simulink model "ROMmodel_C1"  (R2023a)
settings: Solver=ode15s  StartTime=0.0  StopTime=1  RelTol=1e-3
model workspace (MAT data in the model file):
  AbdFlex.InterfaceFrameOrigins = [9.55660193925e-12  0.0959385649697  0.873753152305; 0  0.141459732056  1.16; 0  0.0965046510696  0.917910743713; 0  0.107959739685  0.951083679199; 0  0.115579937935  0.987707740784; 0  0.115174944878  1.02503444672; 0  0.108694094658  1.05941337585]
  AbdFlex.InterfaceFrameOrientations = [0.845190937114  -0.534464479475  0  0; 1  0  0  0; 0.984100197668  -0.177614191295  0  0; 0.981622160978  -0.190834831927  0  0; 0.996532605642  -0.0832031603563  0  0; 0.99971936632  0.0236894196747  0  0; 0.994308117365  0.106542797703  0  0]
  AbdFlex.StiffnessMatrix = [43361.7570519 -71.0199455086 224.354982647 0.636554401137 1595.02399491 -409.551381431 1442.61743363 0.240122053479 21.2711338415 -1.01415324364 563.926777958 237.112118311 ... (1936 elements, 44x44)]
  AbdFlex.MassMatrix = [0.803313954226 0.00157932528245 -0.000141409930888 -6.06093264666e-05 -0.0137464920365 -0.0376787188144 0.0264567110796 0.000154097209329 -0.000731720801434 4.72355458836e-05 -0.0189804601472 -0.0220920974904 ... (1936 elements, 44x44)]
  AbdFlex.GraphicVertexCoordinates = [-0.0249230308533 -0.0239328804016 -0.0255591678619 -0.0472221679687 -0.0482721481323 -0.0496672782898 0.0537743339539 0.053651309967 0.0579661331177 0.00969921779633 0.00303560614586 0.0045106883049 ... (24849 elements, 8283x3)]
  AbdFlex.GraphicFacetVertexIndices = [1 4 7 10 13 16 19 22 25 28 31 34 ... (49686 elements, 16562x3)]
  AbdFlex.GraphicReconstructionMatrix = [-0.0338257388504 0.0155859996042 -0.0281553023712 -0.0314821376202 0.0122493157715 -0.022038070746 -0.0338754415912 0.0120802860785 -0.0268680028964 0.000241192212069 0.0929827996464 -0.0670573257083 ... (1093356 elements, 24849x44)]
  C_IVD = 0.05
  E_AbdFlex = 0.126
  E_IVD1 = 2.1
  E_IVD2 = 2.2
  E_IVD3 = 1.1
  E_IVD4 = 1.7
  E_IVD5 = 3.2
  E_IVD_Optimization : sldodialogs.data.SessionData (value not decoded)
  E_TLFflex = 18.8
  FollowerLoad = 0
  IAP_Pa = 0
  IAP_activation_AbdFlex = 1
  ISL_color = [1 1 1]
  ITL_color = [1 1 1]
  IVD1.InterfaceFrameOrigins = [0.000134062032297  0.0934932940185  1.0361888027; 0.000751265379852  0.0916872213141  1.04242465264]
  IVD1.InterfaceFrameOrientations = [0.998042258458  0.062435296582  -0.00366490237461  -0.000229268114415; 0.994308029006  0.106542788235  0.000419180698234  4.49163428851e-05]
  IVD1.StiffnessMatrix = [58604.0590509 224.692445169 -1325.81371063 11.5597748027 178.44666362 120.502622484 -58604.0590509 -224.69244516 1325.81371063 -10.5664072195 187.81775116 -14.520750133 ... (484 elements, 22x22)]
  IVD1.MassMatrix = [0.00273222555649 2.66777679307e-05 -0.000140988898492 -4.00936120478e-08 -1.20933230549e-06 -3.51698017382e-06 0.00135518459087 -2.57456518557e-05 0.000137551041775 6.02428528449e-08 5.38698497169e-07 -2.32643510543e-06 ... (484 elements, 22x22)]
  IVD1.GraphicVertexCoordinates = [0.0211187839508 0.0199944629669 0.0202089264725 0.0199764213562 0.0218328495026 0.0198232516565 0.019585439682 0.0207308521271 0.0184082126617 0.0186618622796 0.0138851182479 0.018299514437 ... (3174 elements, 1058x3)]
  IVD1.GraphicFacetVertexIndices = [1 4 5 1 8 11 14 17 20 23 26 29 ... (6336 elements, 2112x3)]
  IVD1.GraphicReconstructionMatrix = [0.256410242145 -0.0564519072051 0.218397018955 0.0410678719594 -0.0182233415057 -0.0032254600483 0.0910430192482 -0.0254706457501 0.0263929427812 0.0147481346277 0.0132702076923 0.00432342515969 ... (69828 elements, 3174x22)]
  IVD2.InterfaceFrameOrigins = [0.000686098346355  0.0963570089985  1.0036271702; 0.000140868100677  0.0958234446692  1.01049438711]
  IVD2.InterfaceFrameOrientations = [0.999634432353  -0.0270344006557  -0.000377796940173  1.02172489428e-05; 0.999691730803  0.0236887648214  0.00743335453971  0.000176141286458]
  IVD2.StiffnessMatrix = [56957.407386 -78.2947242504 2352.16731735 -17.870981357 205.105453917 67.0078916822 -56957.407386 78.2947242326 -2352.16731734 17.1536156345 187.3158902 -36.5747621608 ... (484 elements, 22x22)]
  IVD2.MassMatrix = [0.00289222462833 -1.63017581735e-06 -0.000121359011889 5.79569398759e-07 -1.89296391846e-06 -4.55924182037e-06 0.00149269437853 1.27567995597e-06 0.00011497170797 -3.47796966863e-07 -3.11083563762e-07 -3.41019744486e-06 ... (484 elements, 22x22)]
  IVD2.GraphicVertexCoordinates = [0.00590759760452 0.00585390377045 0.00765654303278 0.00585360622406 0.00761780643463 0.00650919151306 -0.0178935775757 -0.0169768047333 -0.0178932800293 -0.0166804516015 0.0200935579478 0.0162637276088 ... (3231 elements, 1077x3)]
  IVD2.GraphicFacetVertexIndices = [1 4 6 7 10 11 14 17 15 22 25 28 ... (6450 elements, 2150x3)]
  IVD2.GraphicReconstructionMatrix = [0 0 0 0 0 0 0 0 0 0.00543110901344 0.00220321782751 -0.000251171527064 ... (71082 elements, 3231x22)]
  IVD3.InterfaceFrameOrigins = [0.000574607735293  0.0917086135207  0.970572111233; 0.000722363834978  0.0932279951662  0.976368556268]
  IVD3.InterfaceFrameOrientations = [0.988186593281  -0.153247753692  -0.00152541347062  0.000236560776491; 0.996532076153  -0.0832031161482  0.00102728042444  -8.57703776093e-05]
  IVD3.StiffnessMatrix = [71304.4780645 62.9837567154 443.050268141 -9.93169999493 256.42256406 64.3509875002 -71304.4780645 -62.9837567061 -443.050268129 10.2397805565 156.824460393 -172.680396477 ... (484 elements, 22x22)]
  IVD3.MassMatrix = [0.00260198817992 -2.70630834152e-05 -0.000116699850829 4.26142700457e-07 -2.89748765883e-06 -5.58917800559e-06 0.00134564423394 2.80094300316e-05 0.000115788329975 -4.23251433064e-07 -1.01057781469e-06 -3.70278721028e-06 ... (484 elements, 22x22)]
  IVD3.GraphicVertexCoordinates = [0.0185354701694 0.0186535515176 0.0152037481739 0.0123575800793 0.0160170742583 -0.0196599748606 -0.0204075508118 -0.0210658721924 -0.00348978233337 -0.00541844558716 -0.00541840410233 0.0207612419128 ... (3219 elements, 1073x3)]
  IVD3.GraphicFacetVertexIndices = [1 3 6 9 12 15 15 19 22 19 26 29 ... (6426 elements, 2142x3)]
  IVD3.GraphicReconstructionMatrix = [0 0 0 0 0 0 0 0 0 0 0 0 ... (70818 elements, 3219x22)]
  IVD4.InterfaceFrameOrigins = [0.000825538133572  0.0809240019453  0.938796986262; 0.000702816801697  0.0842740141936  0.946273348843]
  IVD4.InterfaceFrameOrientations = [0.982784432836  -0.18463310027  0.0066204169361  -0.00124376014022; 0.98161854002  -0.190834127986  -0.00266623568967  0.000518336545308]
  IVD4.StiffnessMatrix = [51887.5634084 863.844390995 1885.99958486 3.3949859294 210.302792214 -101.202544563 -51887.5634085 -863.844390999 -1885.99958487 -3.53527809988 177.858897611 -72.7274405247 ... (484 elements, 22x22)]
  IVD4.MassMatrix = [0.00383476851166 0.000110815534324 0.000274865642717 -1.01453683106e-07 -1.40266595323e-06 1.05697122783e-06 0.00193817912343 -0.00010255217908 -0.000262363391912 1.30237786765e-07 -7.38115151254e-07 4.90959424197e-07 ... (484 elements, 22x22)]
  IVD4.GraphicVertexCoordinates = [-0.00692420768738 -0.00905326843262 -0.00795169639587 0.00511226844788 0.00382958173752 0.00571388053894 0.00635967063904 0.00634820652008 0.00634847593307 0.0207787151337 0.021058713913 0.0196662540436 ... (3462 elements, 1154x3)]
  IVD4.GraphicFacetVertexIndices = [1 4 7 10 13 16 19 22 25 28 31 33 ... (6912 elements, 2304x3)]
  IVD4.GraphicReconstructionMatrix = [0.770337775779 -0.00512670557356 -0.011312131418 0.762650254966 -0.0169645937534 -0.0232414027029 0.996499969395 6.68963973463e-05 -0.000139362803193 0.268412218038 0.0114672418521 0.0636586351525 ... (76164 elements, 3462x22)]
  IVD5.InterfaceFrameOrigins = [0.000376663432785  0.0678773637683  0.904322521845; 0.000371714158635  0.0728931824713  0.914631727172]
  IVD5.InterfaceFrameOrientations = [0.963982062514  -0.265850048581  -0.0076111073418  0.00209901546435; 0.970389572461  -0.241521069818  0.00331223233616  -0.000824384267944]
  IVD5.StiffnessMatrix = [35645.3690223 -248.67083816 -709.947740413 9.43031031091 176.709163243 -84.3584941894 -35645.3690223 248.670838177 709.947740436 -10.4276807361 190.762751227 -94.4309836875 ... (484 elements, 22x22)]
  IVD5.MassMatrix = [0.00473206856693 -8.93206818325e-05 -0.000159656867099 -3.06552159693e-07 -3.25796315086e-07 -2.69868887073e-06 0.00266836567966 8.98622650209e-05 0.000153831007551 3.02118872769e-07 -6.97732994802e-06 7.09837910569e-08 ... (484 elements, 22x22)]
  IVD5.GraphicVertexCoordinates = [0.00965603343984 0.00946946966218 0.00773647180015 -0.000354907704017 0.000199558018267 0.00273271407381 -0.0111632396442 -0.00971436501195 -0.0129904044408 -0.00470198234377 -0.00657061876603 -0.0080616476784 ... (3822 elements, 1274x3)]
  IVD5.GraphicFacetVertexIndices = [1 4 7 10 13 16 19 21 24 26 29 32 ... (7632 elements, 2544x3)]
  IVD5.GraphicReconstructionMatrix = [0 0 0 0 0 0 0 0 0 0 0 0 ... (84084 elements, 3822x22)]
  Left_EO = 0
  Left_IO = 0
  Left_Lat = 18
  Left_LongThor = 0
  Left_MF = 0
  Left_Psoas = 0
  Left_RA = 0
  Moment_AR = 0
  Moment_FE = 7.5
  Moment_LB = 0
  ROM_draft1_sdosession : sldodialogs.data.SessionData (value not decoded)
  ROM_draft3_sdosession : sldodialogs.data.SessionData (value not decoded)
  Right_EO = 0
  Right_IO = 0
  Right_Lat = 18
  Right_LongThor = 0
  Right_MF = 0
  Right_Psoas = 0
  Right_RA = 0
  SSL_color = [1 1 1]
  TLFflex.InterfaceFrameOrigins = [0  0.0286700446606  1.0249522934; 0  0.0299802474976  1.00330503082; 0  0.0254783229828  0.97747177887; 0  0.0207425162792  0.956563842773; 0  0.0144365491867  0.93638368988; 0.049806123159  0.0172518861278  0.922800188881; -0.049806123159  0.0172518861278  0.922800188881; 0.0340383493  -0.010672627765  1.12996748354; 0.0388434691641  -0.00188389440244  1.08212073323; 0.0590475906704  0.0162601057615  1.03264080317; -0.0340383493  -0.010672627765  1.12996748354; -0.0388434691641  -0.00188389440244  1.08212073323; -0.0590475906704  0.0162601057615  1.03264080317]
  TLFflex.InterfaceFrameOrientations = [1  0  0  0; 1  0  0  0; 1  0  0  0; 1  0  0  0; 1  0  0  0; 1  0  0  0; 1  0  0  0; 1  0  0  0; 1  0  0  0; 1  0  0  0; 1  0  0  0; 1  0  0  0; 1  0  0  0]
  TLFflex.StiffnessMatrix = [3066.73590221 18.8871843042 -24.6815054809 0.185355906935 -27.1562999474 -5.00119068721 -2890.55682783 -19.4003823931 24.0128755145 0.225494141951 -25.823043414 2.53663392749 ... (7744 elements, 88x88)]
  TLFflex.MassMatrix = [0.00625522807647 9.49097881264e-06 4.16499662158e-06 -9.78079002209e-08 5.18483232966e-05 -9.11375657364e-07 0.000159214873984 -2.78264603728e-06 -8.02480617259e-06 4.32112453313e-09 2.74154275053e-06 6.17256747742e-06 ... (7744 elements, 88x88)]
  TLFflex.GraphicVertexCoordinates = [-0.0377008171739 -0.0393963165839 -0.0371501493438 0.0614751544791 0.062221073601 0.064095957414 -0.0512719215728 -0.0484790364525 -0.0501779383507 0.0308447883186 0.0321367582765 0.034096923506 ... (26841 elements, 8947x3)]
  TLFflex.GraphicFacetVertexIndices = [1 4 7 10 13 16 19 22 25 28 28 22 ... (53670 elements, 17890x3)]
  TLFflex.GraphicReconstructionMatrix = [0.000464639411671 -0.0178024512743 0.0479271668365 -0.0147231027484 -0.0347333700407 0.045198070726 -0.011349330195 -0.0316574127367 0.0470820475173 -0.00429611905822 0.00365635322465 -0.00297745351186 ... (2362008 elements, 26841x88)]
  TLFflex2.InterfaceFrameOrigins = [0  0.0286700446606  1.0249522934; 0  0.0299802474976  1.00330503082; 0  0.0254783229828  0.97747177887; 0  0.0207425162792  0.956563842773; 0  0.0144365491867  0.93638368988; 0.049806123159  0.0172518861278  0.922800188881; -0.049806123159  0.0172518861278  0.922800188881; 0.0340383493  -0.010672627765  1.12996748354; 0.0388434691641  -0.00188389440244  1.08212073323; 0.0590475906704  0.0162601057615  1.03264080317; -0.0340383493  -0.010672627765  1.12996748354; -0.0388434691641  -0.00188389440244  1.08212073323; -0.0590475906704  0.0162601057615  1.03264080317]
  TLFflex2.InterfaceFrameOrientations = [1  0  0  0; 1  0  0  0; 1  0  0  0; 1  0  0  0; 1  0  0  0; 1  0  0  0; 1  0  0  0; 1  0  0  0; 1  0  0  0; 1  0  0  0; 1  0  0  0; 1  0  0  0; 1  0  0  0]
  TLFflex2.StiffnessMatrix = [3098.16338957 1.85271730265 -2.49344260049 0.0117348050607 -27.4580802755 -4.86875385453 -2927.62857118 -1.89895408806 1.79381618556 0.0403210517395 -26.3931492349 2.34539218598 ... (7744 elements, 88x88)]
  TLFflex2.MassMatrix = [0.00647785700791 -7.69135453041e-06 1.52474405977e-05 -1.66463703749e-08 5.42797116484e-05 -4.88742850574e-07 0.000137311871526 -3.29576441791e-06 3.78371240816e-06 4.33544974799e-09 2.96751088594e-06 6.68238191038e-06 ... (7744 elements, 88x88)]
  TLFflex2.GraphicVertexCoordinates = [0.0187781253155 0.0208486402039 0.0186395397985 0.00960678133698 0.00986427632128 0.0093318262088 0.0122389386043 0.0589722700755 0.0600399895232 0.0577259274432 0.059047073965 0.0568156199971 ... (22956 elements, 7652x3)]
  TLFflex2.GraphicFacetVertexIndices = [1 4 7 8 11 14 17 20 23 26 29 32 ... (45900 elements, 15300x3)]
  TLFflex2.GraphicReconstructionMatrix = [-0.0992460193127 -0.842553190279 -0.169575794245 -0.10048467577 -0.840523805717 -0.167598295688 -0.0880669070773 -0.863197887424 -0.169707154947 -0.0115968837044 0.017512943826 -0.0284024768683 ... (2020128 elements, 22956x88)]
  eo_color = [1 0 0]
  io_color = [0 1 0]
  latissimus_color = [0 0.5 1]
  ligament_radius = 0.5
  longissimus_color = [1 0 1]
  multifidus_color = [0 1 1]
  muscle_radius = 1
  psoas_color = [0 0 1]
  ra_color = [1 1 0.5]
libraries: checkpacklib x11, simulink_extras x9, simulink x2
settings Model: Creator=siril  LastModifiedBy=siril
settings DataIOCC: SaveFormat=StructureWithTime  ReturnWorkspaceOutputsName=SDOSimTest_Log
settings DebuggingCC: UniqueDataStoreMsg=error
settings SimscapeCC: SimscapeLogType=all

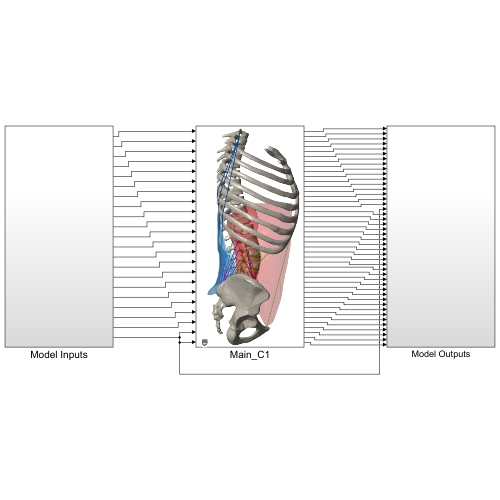
[thumbnail: Simulink's stored preview of the top-level diagram]
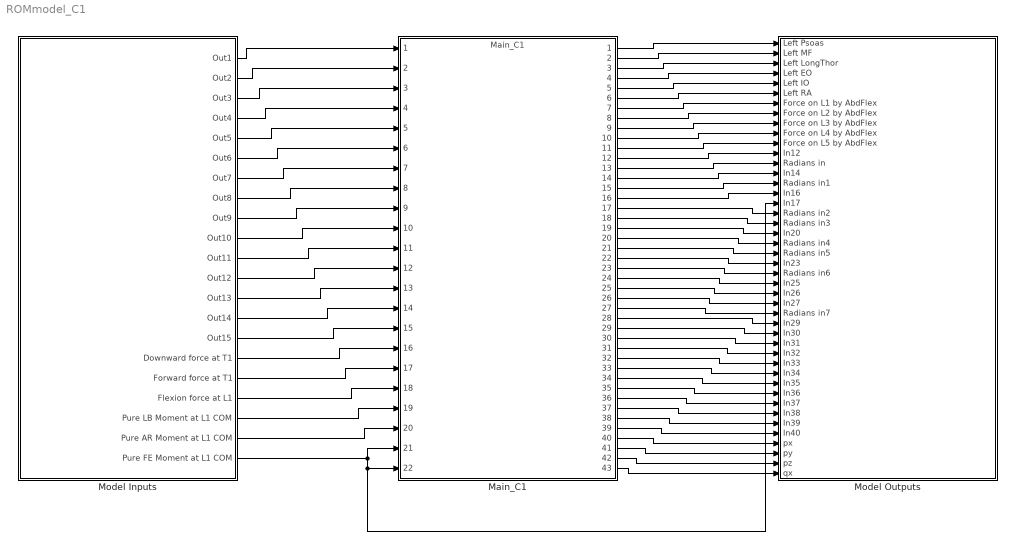
== system ROMmodel_C1 ==
  Main_C1 : ModelReference  ModelNameDialog=Main_C1.slxp  ModelReferenceVersion=1.132  CopyOfModelProtected=on
      mask Display: image('$imagefile'); % Use "Edit Image" on toolstrip to change.
  Model Inputs : Subsystem
  Model Outputs : Subsystem
  wires:
  Main_C1/32 -> Model Outputs/33(In33)
  Main_C1/31 -> Model Outputs/32(In32)
  Model Inputs/20(Pure AR Moment at L1 COM) -> Main_C1/20
  Model Inputs/1(Out1) -> Main_C1/1
  Model Inputs/7(Out7) -> Main_C1/7
  Model Inputs/8(Out8) -> Main_C1/8
  Model Inputs/3(Out3) -> Main_C1/3
  Main_C1/20 -> Model Outputs/21(Radians in4)
  Model Inputs/2(Out2) -> Main_C1/2
  Main_C1/24 -> Model Outputs/25(In25)
  Main_C1/23 -> Model Outputs/24(Radians in6)
  Model Inputs/12(Out12) -> Main_C1/12
  Model Inputs/4(Out4) -> Main_C1/4
  Main_C1/13 -> Model Outputs/13(Radians in)
  Model Inputs/5(Out5) -> Main_C1/5
  Model Inputs/6(Out6) -> Main_C1/6
  Model Inputs/9(Out9) -> Main_C1/9
  Main_C1/19 -> Model Outputs/20(In20)
  Model Inputs/10(Out10) -> Main_C1/10
  Main_C1/22 -> Model Outputs/23(In23)
  Model Inputs/11(Out11) -> Main_C1/11
  Main_C1/25 -> Model Outputs/26(In26)
  Model Inputs/14(Out14) -> Main_C1/14
  Model Inputs/13(Out13) -> Main_C1/13
  Main_C1/26 -> Model Outputs/27(In27)
  Model Inputs/15(Out15) -> Main_C1/15
  Main_C1/28 -> Model Outputs/29(In29)
  Main_C1/27 -> Model Outputs/28(Radians in7)
  Model Inputs/16(Downward force at T1) -> Main_C1/16
  Main_C1/29 -> Model Outputs/30(In30)
  Model Inputs/18(Flexion force at L1) -> Main_C1/18
  Model Inputs/17(Forward force at T1) -> Main_C1/17
  Main_C1/30 -> Model Outputs/31(In31)
  Model Inputs/19(Pure LB Moment at L1 COM) -> Main_C1/19
  Main_C1/33 -> Model Outputs/34(In34)
  Model Inputs/21(Pure FE Moment at L1 COM) -> Model Outputs/17(In17), Main_C1/22, Main_C1/21
  Main_C1/1 -> Model Outputs/1(Left Psoas)
  Main_C1/2 -> Model Outputs/2(Left MF)
  Main_C1/3 -> Model Outputs/3(Left LongThor)
  Main_C1/4 -> Model Outputs/4(Left EO)
  Main_C1/5 -> Model Outputs/5(Left IO)
  Main_C1/6 -> Model Outputs/6(Left RA)
  Main_C1/7 -> Model Outputs/7(Force on L1 by AbdFlex)
  Main_C1/8 -> Model Outputs/8(Force on L2 by AbdFlex)
  Main_C1/9 -> Model Outputs/9(Force on L3 by AbdFlex)
  Main_C1/10 -> Model Outputs/10(Force on L4 by AbdFlex)
  Main_C1/11 -> Model Outputs/11(Force on L5 by AbdFlex)
  Main_C1/12 -> Model Outputs/12(In12)
  Main_C1/14 -> Model Outputs/14(In14)
  Main_C1/15 -> Model Outputs/15(Radians in1)
  Main_C1/16 -> Model Outputs/16(In16)
  Main_C1/17 -> Model Outputs/18(Radians in2)
  Main_C1/18 -> Model Outputs/19(Radians in3)
  Main_C1/21 -> Model Outputs/22(Radians in5)
  Main_C1/34 -> Model Outputs/35(In35)
  Main_C1/36 -> Model Outputs/37(In37)
  Main_C1/35 -> Model Outputs/36(In36)
  Main_C1/37 -> Model Outputs/38(In38)
  Main_C1/38 -> Model Outputs/39(In39)
  Main_C1/40 -> Model Outputs/41(px)
  Main_C1/39 -> Model Outputs/40(In40)
  Main_C1/41 -> Model Outputs/42(py)
  Main_C1/42 -> Model Outputs/43(pz)
  Main_C1/43 -> Model Outputs/44(qx)

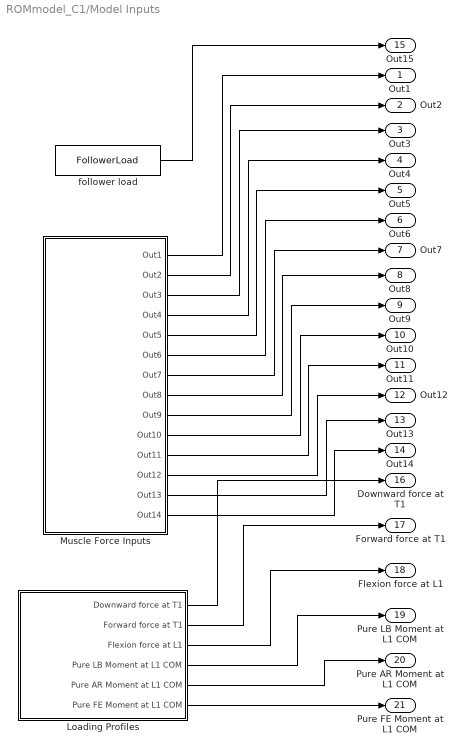
== system ROMmodel_C1/Model Inputs ==
  Loading Profiles : Subsystem
  Muscle Force Inputs : Subsystem
  follower load : Constant  Value=FollowerLoad
  Out1 : Outport
  Out2 : Outport  Port=2
  Out3 : Outport  Port=3
  Out4 : Outport  Port=4
  Out5 : Outport  Port=5
  Out6 : Outport  Port=6
  Out7 : Outport  Port=7
  Out8 : Outport  Port=8
  Out9 : Outport  Port=9
  Out10 : Outport  Port=10
  Out11 : Outport  Port=11
  Out12 : Outport  Port=12
  Out13 : Outport  Port=13
  Out14 : Outport  Port=14
  Out15 : Outport  Port=15
  Downward force at T1 : Outport  Port=16
  Forward force at T1 : Outport  Port=17
  Flexion force at L1 : Outport  Port=18
  Pure LB Moment at L1 COM : Outport  Port=19
  Pure AR Moment at L1 COM : Outport  Port=20
  Pure FE Moment at L1 COM : Outport  Port=21
  wires:
  Muscle Force Inputs/12(Out12) -> Out12/1
  follower load/1 -> Out15/1
  Loading Profiles/6(Pure FE Moment at L1 COM) -> Pure FE Moment at L1 COM/1
  Loading Profiles/3(Flexion force at L1) -> Flexion force at L1/1
  Muscle Force Inputs/1(Out1) -> Out1/1
  Muscle Force Inputs/2(Out2) -> Out2/1
  Muscle Force Inputs/5(Out5) -> Out5/1
  Muscle Force Inputs/6(Out6) -> Out6/1
  Muscle Force Inputs/3(Out3) -> Out3/1
  Muscle Force Inputs/4(Out4) -> Out4/1
  Muscle Force Inputs/7(Out7) -> Out7/1
  Muscle Force Inputs/8(Out8) -> Out8/1
  Muscle Force Inputs/10(Out10) -> Out10/1
  Muscle Force Inputs/9(Out9) -> Out9/1
  Loading Profiles/4(Pure LB Moment at L1 COM) -> Pure LB Moment at L1 COM/1
  Muscle Force Inputs/11(Out11) -> Out11/1
  Muscle Force Inputs/13(Out13) -> Out13/1
  Muscle Force Inputs/14(Out14) -> Out14/1
  Loading Profiles/1(Downward force at T1) -> Downward force at T1/1
  Loading Profiles/2(Forward force at T1) -> Forward force at T1/1
  Loading Profiles/5(Pure AR Moment at L1 COM) -> Pure AR Moment at L1 COM/1

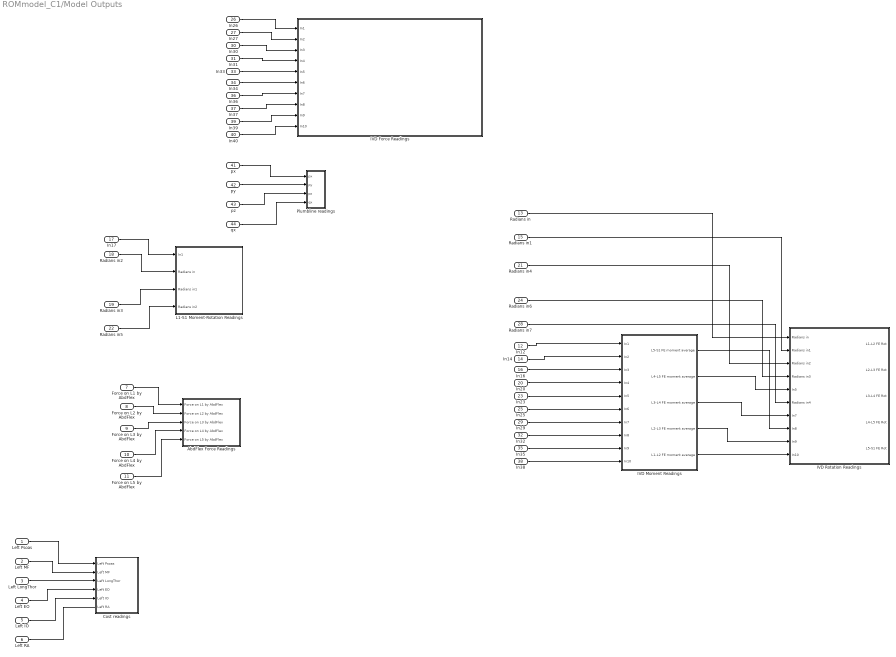
== system ROMmodel_C1/Model Outputs ==
  Left Psoas : Inport
  Left MF : Inport  Port=2
  Left LongThor : Inport  Port=3
  Left EO : Inport  Port=4
  Left IO : Inport  Port=5
  Left RA : Inport  Port=6
  Force on L1 by AbdFlex : Inport  Port=7
  Force on L2 by AbdFlex : Inport  Port=8
  Force on L3 by AbdFlex : Inport  Port=9
  Force on L4 by AbdFlex : Inport  Port=10
  Force on L5 by AbdFlex : Inport  Port=11
  In12 : Inport  Port=12
  Radians in : Inport  Port=13
  In14 : Inport  Port=14
  Radians in1 : Inport  Port=15
  In16 : Inport  Port=16
  In17 : Inport  Port=17
  Radians in2 : Inport  Port=18
  Radians in3 : Inport  Port=19
  In20 : Inport  Port=20
  Radians in4 : Inport  Port=21
  Radians in5 : Inport  Port=22
  In23 : Inport  Port=23
  Radians in6 : Inport  Port=24
  In25 : Inport  Port=25
  In26 : Inport  Port=26
  In27 : Inport  Port=27
  Radians in7 : Inport  Port=28
  In29 : Inport  Port=29
  In30 : Inport  Port=30
  In31 : Inport  Port=31
  In32 : Inport  Port=32
  In33 : Inport  Port=33
  In34 : Inport  Port=34
  In35 : Inport  Port=35
  In36 : Inport  Port=36
  In37 : Inport  Port=37
  In38 : Inport  Port=38
  In39 : Inport  Port=39
  In40 : Inport  Port=40
  px : Inport  Port=41
  py : Inport  Port=42
  pz : Inport  Port=43
  qx : Inport  Port=44
  AbdFlex Force Readings : Subsystem
  Cost readings : Subsystem
  IVD Force Readings : Subsystem
  IVD Moment Readings : Subsystem
  IVD Rotation Readings : Subsystem
  L1-S1 Moment-Rotation Readings : Subsystem
  Plumbline readings : Subsystem
  wires:
  Left MF/1 -> Cost readings/2(Left MF)
  In37/1 -> IVD Force Readings/8(In8)
  In26/1 -> IVD Force Readings/1(In1)
  Radians in7/1 -> IVD Rotation Readings/6(Radians in4)
  In27/1 -> IVD Force Readings/2(In2)
  In30/1 -> IVD Force Readings/3(In3)
  In32/1 -> IVD Moment Readings/8(In8)
  In31/1 -> IVD Force Readings/4(In4)
  In33/1 -> IVD Force Readings/5(In5)
  In34/1 -> IVD Force Readings/6(In6)
  In35/1 -> IVD Moment Readings/9(In9)
  In36/1 -> IVD Force Readings/7(In7)
  Left EO/1 -> Cost readings/4(Left EO)
  In40/1 -> IVD Force Readings/10(In10)
  In39/1 -> IVD Force Readings/9(In9)
  px/1 -> Plumbline readings/1(px)
  py/1 -> Plumbline readings/2(py)
  qx/1 -> Plumbline readings/4(qx)
  pz/1 -> Plumbline readings/3(pz)
  Left Psoas/1 -> Cost readings/1(Left Psoas)
  Left LongThor/1 -> Cost readings/3(Left LongThor)
  Left IO/1 -> Cost readings/5(Left IO)
  Force on L1 by AbdFlex/1 -> AbdFlex Force Readings/1(Force on L1 by AbdFlex)
  Left RA/1 -> Cost readings/6(Left RA)
  In12/1 -> IVD Moment Readings/1(In1)
  Radians in1/1 -> IVD Rotation Readings/2(Radians in1)
  In14/1 -> IVD Moment Readings/2(In2)
  In16/1 -> IVD Moment Readings/3(In3)
  In20/1 -> IVD Moment Readings/4(In4)
  Radians in5/1 -> L1-S1 Moment-Rotation Readings/4(Radians in2)
  In23/1 -> IVD Moment Readings/5(In5)
  In25/1 -> IVD Moment Readings/6(In6)
  In29/1 -> IVD Moment Readings/7(In7)
  In38/1 -> IVD Moment Readings/10(In10)
  IVD Moment Readings/1(L5-S1 FE moment average) -> IVD Rotation Readings/8(In8)
  Radians in/1 -> IVD Rotation Readings/1(Radians in)
  In17/1 -> L1-S1 Moment-Rotation Readings/1(In1)
  Radians in3/1 -> L1-S1 Moment-Rotation Readings/3(Radians in1)
  Radians in2/1 -> L1-S1 Moment-Rotation Readings/2(Radians in)
  Radians in4/1 -> IVD Rotation Readings/3(Radians in2)
  Radians in6/1 -> IVD Rotation Readings/4(Radians in3)
  Force on L2 by AbdFlex/1 -> AbdFlex Force Readings/2(Force on L2 by AbdFlex)
  Force on L3 by AbdFlex/1 -> AbdFlex Force Readings/3(Force on L3 by AbdFlex)
  Force on L5 by AbdFlex/1 -> AbdFlex Force Readings/5(Force on L5 by AbdFlex)
  Force on L4 by AbdFlex/1 -> AbdFlex Force Readings/4(Force on L4 by AbdFlex)
  IVD Moment Readings/2(L4-L5 FE moment average) -> IVD Rotation Readings/5(In5)
  IVD Moment Readings/3(L3-L4 FE moment average) -> IVD Rotation Readings/7(In7)
  IVD Moment Readings/4(L2-L3 FE moment average) -> IVD Rotation Readings/9(In9)
  IVD Moment Readings/5(L1-L2 FE moment average) -> IVD Rotation Readings/10(In10)

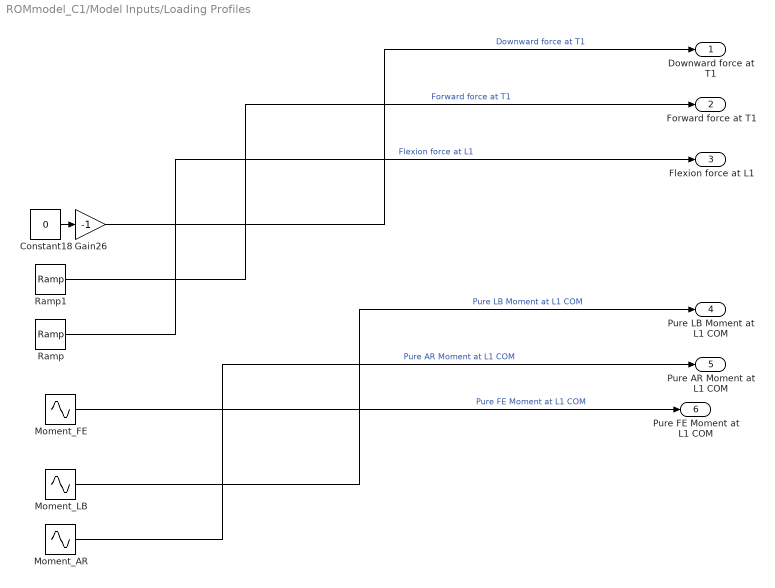
== system ROMmodel_C1/Model Inputs/Loading Profiles ==
  Constant18 : Constant  Value=0
  Gain26 : Gain  Gain=-1
  Moment_AR : Sin  Amplitude=Moment_AR  Frequency=pi*0.5  SampleTime=0
  Moment_FE : Sin  Amplitude=-Moment_FE  Frequency=pi*0.5  SampleTime=0
  Moment_LB : Sin  Amplitude=-Moment_LB  Frequency=pi*0.5  SampleTime=0
  Ramp : simulink/Sources/Ramp  slope=0  start=0  InitialOutput=0
  Ramp1 : simulink/Sources/Ramp  slope=0  start=0  InitialOutput=0
  Downward force at T1 : Outport
  Forward force at T1 : Outport  Port=2
  Flexion force at L1 : Outport  Port=3
  Pure LB Moment at L1 COM : Outport  Port=4
  Pure AR Moment at L1 COM : Outport  Port=5
  Pure FE Moment at L1 COM : Outport  Port=6
  wires:
  Ramp1/1 -> Forward force at T1/1  "Forward force at T1"
  Moment_AR/1 -> Pure AR Moment at L1 COM/1  "Pure AR Moment at L1 COM"
  Constant18/1 -> Gain26/1
  Ramp/1 -> Flexion force at L1/1  "Flexion force at L1"
  Moment_LB/1 -> Pure LB Moment at L1 COM/1  "Pure LB Moment at L1 COM"
  Moment_FE/1 -> Pure FE Moment at L1 COM/1  "Pure FE Moment at L1 COM"
  Gain26/1 -> Downward force at T1/1  "Downward force at T1"

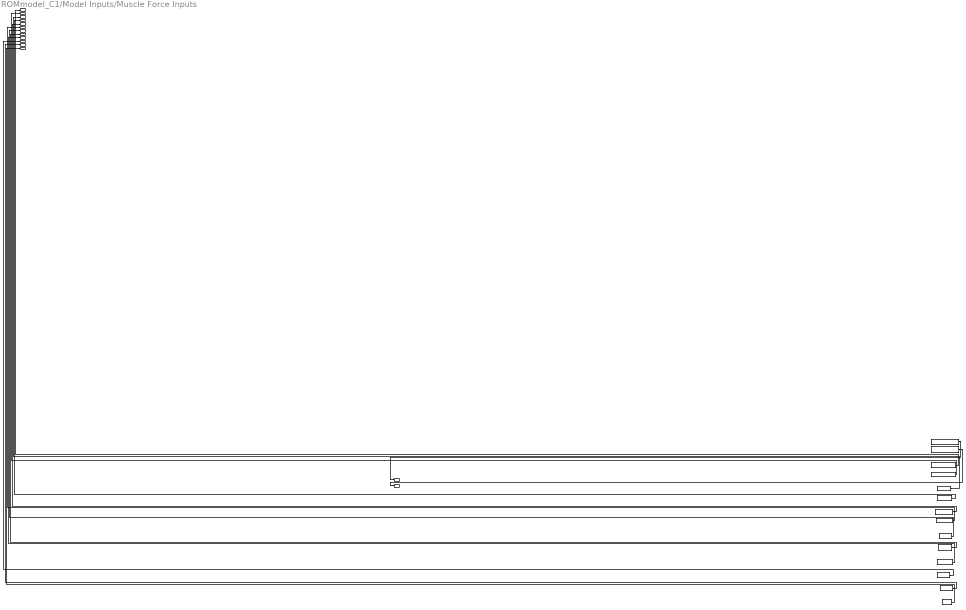
== system ROMmodel_C1/Model Inputs/Muscle Force Inputs ==
  Constant2 : Constant  Value=Right_Lat
  Constant3 : Constant  Value=Left_Lat
  Left_EO : Constant  Value=Left_EO
  Left_IO : Constant  Value=Left_IO
  Left_LongThor : Constant  Value=Left_LongThor
  Left_MF : Constant  Value=Left_MF
  Left_Psoas : Constant  Value=Left_Psoas
  Left_RA : Constant  Value=Left_RA
  Right EO : Constant  Value=Right_EO
  Right IO : Constant  Value=Right_IO
  Right LongThor : Constant  Value=Right_LongThor
  Right MF : Constant  Value=Right_MF
  Right Psoas : Constant  Value=Right_Psoas
  Right RA : Constant  Value=Right_RA
  Out1 : Outport
  Out2 : Outport  Port=2
  Out3 : Outport  Port=3
  Out4 : Outport  Port=4
  Out5 : Outport  Port=5
  Out6 : Outport  Port=6
  Out7 : Outport  Port=7
  Out8 : Outport  Port=8
  Out9 : Outport  Port=9
  Out10 : Outport  Port=10
  Out11 : Outport  Port=11
  Out12 : Outport  Port=12
  Out13 : Outport  Port=13
  Out14 : Outport  Port=14
  wires:
  Right MF/1 -> Out3/1
  Right IO/1 -> Out9/1
  Left_EO/1 -> Out8/1
  Constant3/1 -> Out14/1
  Constant2/1 -> Out13/1
  Right EO/1 -> Out7/1
  Right LongThor/1 -> Out5/1
  Left_LongThor/1 -> Out6/1
  Right Psoas/1 -> Out1/1
  Left_RA/1 -> Out12/1
  Left_IO/1 -> Out10/1
  Right RA/1 -> Out11/1
  Left_MF/1 -> Out4/1
  Left_Psoas/1 -> Out2/1

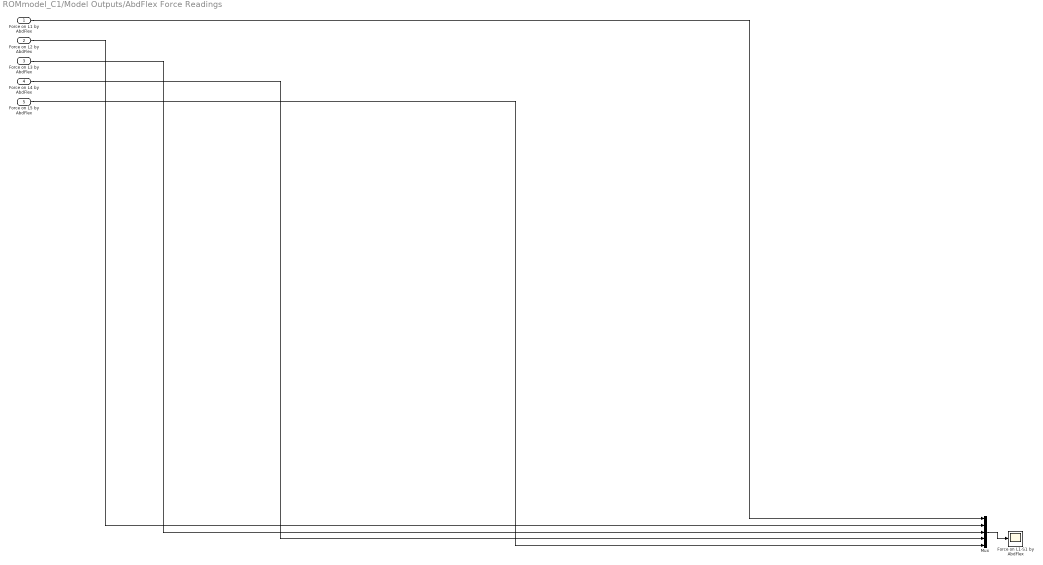
== system ROMmodel_C1/Model Outputs/AbdFlex Force Readings ==
  Force on L1 by AbdFlex : Inport
  Force on L2 by AbdFlex : Inport  Port=2
  Force on L3 by AbdFlex : Inport  Port=3
  Force on L4 by AbdFlex : Inport  Port=4
  Force on L5 by AbdFlex : Inport  Port=5
  Force on L1-S1 by AbdFlex : Scope
  Mux : Mux  Inputs=5
  wires:
  Mux/1 -> Force on L1-S1 by AbdFlex/1
  Force on L4 by AbdFlex/1 -> Mux/4
  Force on L3 by AbdFlex/1 -> Mux/3
  Force on L5 by AbdFlex/1 -> Mux/5
  Force on L2 by AbdFlex/1 -> Mux/2
  Force on L1 by AbdFlex/1 -> Mux/1

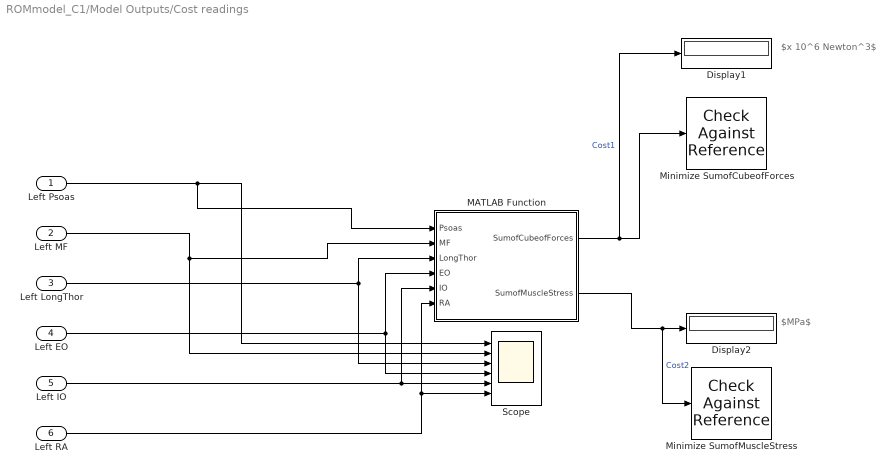
== system ROMmodel_C1/Model Outputs/Cost readings ==
  note: $x 10^6 Newton^3$
  note: $MPa$
  Left Psoas : Inport
  Left MF : Inport  Port=2
  Left LongThor : Inport  Port=3
  Left EO : Inport  Port=4
  Left IO : Inport  Port=5
  Left RA : Inport  Port=6
  Display1 : Display
  Display2 : Display
  MATLAB Function : MATLAB Function
  Minimize SumofCubeofForces : checkpacklib/Check Against Reference  InstantiateOnLoad=on  enabled=off  stopWhenAssertionFail=off  export=off  ViewDlgPos=[360 309 510 390]  OpenViewOnLoad=off  LaunchViewOnOpen=off  TimeUnits=seconds  EnableReferenceBound=on  AbsTolerance=0  RelTolerance=0  ReferenceTimes=[0 1]  ReferenceAmplitudes=[0 0];
      settings: LibrarySourceBlock=sdolib/Signal Constraints/Check Against Reference
  Minimize SumofMuscleStress : checkpacklib/Check Against Reference  InstantiateOnLoad=on  enabled=off  stopWhenAssertionFail=off  export=off  ViewDlgPos=[360 309 510 390]  OpenViewOnLoad=off  LaunchViewOnOpen=off  TimeUnits=seconds  EnableReferenceBound=on  AbsTolerance=0  RelTolerance=0  ReferenceTimes=[0 1]  ReferenceAmplitudes=[0 0];
      settings: LibrarySourceBlock=sdolib/Signal Constraints/Check Against Reference
  Scope : Scope  NumInputPorts=6
  wires:
  MATLAB Function/2(SumofMuscleStress) -> Minimize SumofMuscleStress/1, Display2/1  "Cost2"
  Left EO/1 -> Scope/4, MATLAB Function/4(EO)
  Left RA/1 -> Scope/6, MATLAB Function/6(RA)
  Left IO/1 -> Scope/5, MATLAB Function/5(IO)
  Left MF/1 -> MATLAB Function/2(MF), Scope/2
  Left Psoas/1 -> MATLAB Function/1(Psoas), Scope/1
  Left LongThor/1 -> Scope/3, MATLAB Function/3(LongThor)
  MATLAB Function/1(SumofCubeofForces) -> Minimize SumofCubeofForces/1, Display1/1  "Cost1"

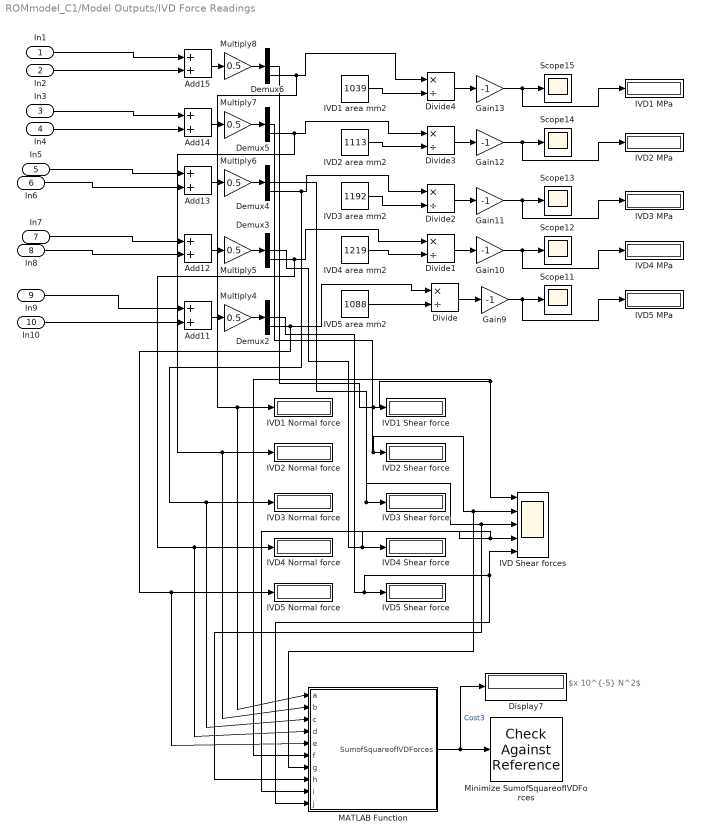
== system ROMmodel_C1/Model Outputs/IVD Force Readings ==
  note: $x 10^{-5} N^2$
  In1 : Inport
  In2 : Inport  Port=2
  In3 : Inport  Port=3
  In4 : Inport  Port=4
  In5 : Inport  Port=5
  In6 : Inport  Port=6
  In7 : Inport  Port=7
  In8 : Inport  Port=8
  In9 : Inport  Port=9
  In10 : Inport  Port=10
  Add{11..15} (5 alike) : Sum
  Demux{2..6} (5 alike) : Demux  Outputs=3
  Display7 : Display
  Divide{,1..4} (5 alike) : Product  Inputs=*/
  Gain{9..13} (5 alike) : Gain  Gain=-1
  IVD Shear forces : Scope  NumInputPorts=5
  IVD1 MPa : Display
  IVD1 Normal force : Display
  IVD1 Shear force : Display
  IVD1 area mm2 : Constant  Value=1039
  IVD2 MPa : Display
  IVD2 Normal force : Display
  IVD2 Shear force : Display
  IVD2 area mm2 : Constant  Value=1113
  IVD3 MPa : Display
  IVD3 Normal force : Display
  IVD3 Shear force : Display
  IVD3 area mm2 : Constant  Value=1192
  IVD4 MPa : Display
  IVD4 Normal force : Display
  IVD4 Shear force : Display
  IVD4 area mm2 : Constant  Value=1219
  IVD5 MPa : Display
  IVD5 Normal force : Display
  IVD5 Shear force : Display
  IVD5 area mm2 : Constant  Value=1088
  MATLAB Function : MATLAB Function  TreatAsAtomicUnit=on
  Minimize SumofSquareofIVDForces : checkpacklib/Check Against Reference  InstantiateOnLoad=on  enabled=off  stopWhenAssertionFail=off  export=off  ViewDlgPos=[360 309 510 390]  OpenViewOnLoad=off  LaunchViewOnOpen=off  TimeUnits=seconds  EnableReferenceBound=on  AbsTolerance=0  RelTolerance=0  ReferenceTimes=[0 1]  ReferenceAmplitudes=[0 0];
      settings: LibrarySourceBlock=sdolib/Signal Constraints/Check Against Reference
  Multiply{4..8} (5 alike) : Gain  Gain=0.5
  Scope{11..15} (5 alike) : Scope
  wires:
  Gain12/1 -> IVD2 MPa/1, Scope14/1
  Multiply6/1 -> Demux4/1
  Demux2/3 -> MATLAB Function/5(e), IVD5 Normal force/1, Divide/1
  Demux3/2 -> MATLAB Function/9(i), IVD Shear forces/4, IVD4 Shear force/1
  Demux5/3 -> MATLAB Function/2(b), IVD2 Normal force/1, Divide3/1
  Add13/1 -> Multiply6/1
  Multiply7/1 -> Demux5/1
  Divide1/1 -> Gain10/1
  Demux5/2 -> IVD Shear forces/2, MATLAB Function/7(g), IVD2 Shear force/1
  IVD4 area mm2/1 -> Divide1/2
  Add14/1 -> Multiply7/1